simulink model "TosAeb"  (R2021a)
settings: Solver=FixedStepDiscrete  StartTime=0.0  StopTime=inf  FixedStep=0.01  RelTol=1e-3
undefined symbols (not defined in the file or in its folder): ACCModSts, AccSts1, AEB_ObjectMovingOrientation, AEB_ObjectMovingState, CN_BTCActive, CN_CurrentGearR, CN_CurrentGearR_DHT, CN_PTCActive, CN_VDCActive, CN_VSC_EspOff, CN_VSC_FCW_AEB_Error, CN_VSC_FCW_AEB_NoDispay, CN_VSC_FCW_AEB_PerfmDgdtn, CN_VSC_FCW_AEB_TempUnAvlbl, CN_VSC_VehStandstill, CNaccelAvoidMax, CNaccelAvoidMin, CNbrakePedalPress, CNcomfortStrLeftAccelMax, CNcomfortStrLeftAccelMin, CNcomfortStrRightAccelMax, CNcomfortStrRightAccelMin, CNDrvDoorOpen, CNDrvModeEco, CNDrvModeNorm, CNDrvModeOther, CNDrvModeSport, CNDrvSBRreminder, CNNull, CNobjInvalidID, CNPassDoorOpen, CNPassSBRreminder, CNPEBSAccelNegInf, CNPEBSAccelNull, CNPEBSTimeInf, CNPEBSTimeNull, CNSTOPMAX, CNSTOPNULL, CNsubjWidth, CNSwtOFF, CNSwtON, CNTTCMAX, CNTTCNULL, CNVehConfigAEBCycNone, CNVehConfigAEBNone, CNVehConfigAEBPedNone, CNVehConfigFCWNone, CNVehConfigJANone, CollisionSeverity, FALSE, FCWSnvty, JACycObjCalcInfoDefaultParams, JAPedObjCalcInfoDefaultParams, JAVehObjCalcInfoDefaultParams, latCycObjCalcInfoDefaultParams, latPedObjCalcInfoDefaultParams, longObjCalcInfoDefaultParams, ModSts, movingStatus, ObjectType ... (+1112)
libraries: simulink x3031, AEBSFunctionLib x50, Ta_Library x33, AEBSStateMachineLib x23, Mylibrary x12, simulink_extras x7, ObjSelect_lib x3, dspstat3 x2
(part 51 of 102)

== system TosAeb/AEB_Objsel/TA_Processor/PreProcessor/ObjectPrecessor/TAObjInfoCalc/For Each Subsystem/Info_Check1/Basic_Info_check/IDProcess ==
  ID_change : Inport
  ObsID_u8 : Inport  Port=2
  Compare To Constant : simulink/Logic and Bit Operations/Compare To Constant  relop===  const=1  OutDataTypeStr=boolean
  Compare To Constant1 : simulink/Logic and Bit Operations/Compare To Constant  relop=~=  const=0  OutDataTypeStr=boolean
  Constant : Constant  OutDataTypeStr=uint8
  Constant1 : Constant  Value=0  OutDataTypeStr=uint8
  Logical Operator : Logic  Operator=OR  AllPortsSameDT=off  OutDataTypeStr=boolean
  Switch : Switch  Criteria=u2 > Threshold
  Obs_ID : Outport
  wires:
  ID_change/1 -> Compare To Constant/1
  ObsID_u8/1 -> Compare To Constant1/1
  Compare To Constant/1 -> Logical Operator/1
  Compare To Constant1/1 -> Logical Operator/2
  Logical Operator/1 -> Switch/2
  Switch/1 -> Obs_ID/1
  Constant/1 -> Switch/1
  Constant1/1 -> Switch/3

== system TosAeb/AEB_Objsel/TA_Processor/PreProcessor/ObjectPrecessor/TAObjInfoCalc/For Each Subsystem/Info_Check1/Basic_Info_check/QuaCorrect ==
  Obj_ID : Inport
  ObsConfidence_u8 : Inport  Port=2
  Veh_AEBFunState : Inport  Port=3
  dy_flg : Inport  Port=4
  dx_flg : Inport  Port=5
  vx_flg : Inport  Port=6
  vy_flg : Inport  Port=7
  If1 : If
  Merge : Merge
  Subsystem : Subsystem
  quality_recrectionelse : Subsystem
  quality_recrectionif : Subsystem  TreatAsAtomicUnit=on
  Fus_ObjQuality : Outport
  wires:
  Merge/1 -> Fus_ObjQuality/1
  quality_recrectionelse/1(Fus_ObjQuality) -> Merge/2
  quality_recrectionif/1(Fus_ObjQuality) -> Merge/1
  If1/1 -> quality_recrectionif/Action
  If1/2 -> quality_recrectionelse/Action
  vy_flg/1 -> quality_recrectionelse/6(vy_flg)
  vx_flg/1 -> quality_recrectionelse/5(vx_flg)
  dx_flg/1 -> quality_recrectionelse/4(dx_flg)
  dy_flg/1 -> quality_recrectionelse/3(dy_flg)
  Veh_AEBFunState/1 -> quality_recrectionelse/2(Veh_AEBFunState)
  ObsConfidence_u8/1 -> quality_recrectionif/1(Fus_TargetQuality), quality_recrectionelse/1(Fus_TargetQuality)
  Subsystem/1(Obj_Invalid) -> If1/1
  Obj_ID/1 -> Subsystem/1(Obj_ID)

== system TosAeb/AEB_Objsel/TA_Processor/PreProcessor/ObjectPrecessor/TAObjInfoCalc/For Each Subsystem/Info_Check1/Basic_Info_check/VxCorrect ==
  Obj_ID : Inport
  Fus_ObjVx_Single : Inport  Port=2
  Fus_ObjVxStd : Inport  Port=3
  Fus_ObjDx_Single : Inport  Port=4
  Veh_AEBFunState : Inport  Port=5
  If : If
  If Action Subsystem : Subsystem  TreatAsAtomicUnit=on
  Merge : Merge
  Merge3 : Merge
  Subsystem : Subsystem
  Subsystem3 : Subsystem
  Fus_ObjVx : Outport
  Vx_CorrectFlg : Outport  Port=2
  wires:
  Merge3/1 -> Vx_CorrectFlg/1
  Subsystem3/2(Vx_CorrectFlg) -> Merge3/2
  If Action Subsystem/2(Vx_CorrectFlg) -> Merge3/1
  Merge/1 -> Fus_ObjVx/1
  Subsystem3/1(Fus_ObjVx) -> Merge/2
  If Action Subsystem/1(Fus_ObjVx) -> Merge/1
  If/2 -> Subsystem3/Action
  Veh_AEBFunState/1 -> Subsystem3/4(Veh_AEBFunState)
  Fus_ObjVxStd/1 -> Subsystem3/3(Fus_ObjVxStd)
  Fus_ObjDx_Single/1 -> Subsystem3/2(Fus_ObjDx_Single)
  Fus_ObjVx_Single/1 -> If Action Subsystem/1(Fus_ObjVx_Single), Subsystem3/1(Fus_ObjVx_Single)
  If/1 -> If Action Subsystem/Action
  Obj_ID/1 -> Subsystem/1(Obj_ID)
  Subsystem/1(Obj_Invalid) -> If/1

== system TosAeb/AEB_Objsel/TA_Processor/PreProcessor/ObjectPrecessor/TAObjInfoCalc/For Each Subsystem/Info_Check1/Basic_Info_check/VyCorrect ==
  Obj_ID : Inport
  Fus_ObjVy_Single : Inport  Port=2
  Fus_TargetState : Inport  Port=3
  Veh_YawRate : Inport  Port=4
  Fus_ObsVyStd : Inport  Port=5
  Fus_ObjDy_Single : Inport  Port=6
  Veh_AEBFunState : Inport  Port=7
  If : If
  If Action Subsystem : Subsystem  TreatAsAtomicUnit=on
  Merge : Merge
  Merge3 : Merge
  Subsystem : Subsystem
  Subsystem3 : Subsystem
  Fus_ObjVy : Outport
  Vy_CorrectFlg : Outport  Port=2
  wires:
  Fus_TargetState/1 -> Subsystem3/4(Fus_TargetState)
  Subsystem3/2(Vy_CorrectFlg) -> Merge3/2
  Merge3/1 -> Vy_CorrectFlg/1
  If Action Subsystem/2(Vy_CorrectFlg) -> Merge3/1
  Subsystem3/1(Fus_ObjVy) -> Merge/2
  Merge/1 -> Fus_ObjVy/1
  If Action Subsystem/1(Fus_ObjVy) -> Merge/1
  If/2 -> Subsystem3/Action
  If/1 -> If Action Subsystem/Action
  Veh_AEBFunState/1 -> Subsystem3/3(Veh_AEBFunState)
  Fus_ObjDy_Single/1 -> Subsystem3/2(Fus_ObjDy_Single)
  Fus_ObjVy_Single/1 -> If Action Subsystem/1(Fus_ObjVy_Single), Subsystem3/1(Fus_ObjVy_Single)
  Obj_ID/1 -> Subsystem/1(Obj_ID)
  Subsystem/1(Obj_Invalid) -> If/1
  Fus_ObsVyStd/1 -> Subsystem3/6(Fus_ObsVyStd)
  Veh_YawRate/1 -> Subsystem3/5(Veh_YawRate)

== system TosAeb/AEB_Objsel/TA_Processor/PreProcessor/ObjectPrecessor/TAObjInfoCalc/For Each Subsystem/Info_Check1/ID_check/IDValid_check ==
  note: ObsConfidence_u8:
      | 				 根据CNN检测计算出的障碍物存在
      | 				 概率，当连续>6时，意味着高conf
      | 				障碍物目标。
  note: ObsAge_u16:
      | 			这个障碍的帧龄是存在的，因为一些后
      | 			处理逻辑来确保跟踪状态和置信度，
      | 			在第一帧中可能不是“1”
  note: ObsValid_u8 :
      | 			0=invalid Obstacle(default value)
      | 			1=new valid (detected this frame)
      | 			2=older valid
  ObsID_u8 : Inport
  ObsValid_u8 : Inport  Port=2
  ObsAge_u16 : Inport  Port=3
  ObsConfidence_u8 : Inport  Port=4
  Action Port : ActionPort  ActionPortLabel=else
  Compare To Constant : simulink/Logic and Bit Operations/Compare To Constant  relop=>=  const=1  OutDataTypeStr=boolean
  Compare To Constant1 : simulink/Logic and Bit Operations/Compare To Constant  relop=>=  const=1  OutDataTypeStr=boolean
  Compare To Constant2 : simulink/Logic and Bit Operations/Compare To Constant  relop=>=  const=6  OutDataTypeStr=boolean
  Constant : Constant  Value=0  OutDataTypeStr=uint8
  Logical Operator : Logic  AllPortsSameDT=off  OutDataTypeStr=boolean
  Logical Operator1 : Logic  Operator=NOT  AllPortsSameDT=off  OutDataTypeStr=boolean
  Switch : Switch  Criteria=u2 ~= 0
  Terminator : Terminator
  Fus_ObjID : Outport
  ID_Change : Outport  Port=2
  wires:
  ObsValid_u8/1 -> Compare To Constant/1
  ObsAge_u16/1 -> Compare To Constant1/1
  ObsConfidence_u8/1 -> Compare To Constant2/1
  Compare To Constant/1 -> Logical Operator/1
  Compare To Constant1/1 -> Logical Operator/2
  Logical Operator/1 -> Switch/2, Logical Operator1/1
  ObsID_u8/1 -> Switch/1
  Constant/1 -> Switch/3
  Switch/1 -> Fus_ObjID/1
  Logical Operator1/1 -> ID_Change/1
  Compare To Constant2/1 -> Terminator/1

== system TosAeb/AEB_Objsel/TA_Processor/PreProcessor/ObjectPrecessor/TAObjInfoCalc/For Each Subsystem/Info_Check1/ID_check/ID_Judge ==
  ObsID_u8 : Inport
  Compare To Constant : simulink/Logic and Bit Operations/Compare To Constant  relop===  const=0  OutDataTypeStr=boolean
  ID_Invalid : Outport
  wires:
  ObsID_u8/1 -> Compare To Constant/1
  Compare To Constant/1 -> ID_Invalid/1

== system TosAeb/AEB_Objsel/TA_Processor/PreProcessor/ObjectPrecessor/TAObjInfoCalc/For Each Subsystem/Info_Check1/ID_check/Invalid ==
  ObsID_u8 : Inport
  Action Port : ActionPort  ActionPortLabel=if(u1 == 1)
  Constant : Constant  Value=0  OutDataTypeStr=boolean
  Signal Conversion : SignalConversion  OverrideOpt=off
  Fus_ObjID : Outport
  ID_Change : Outport  Port=2
  wires:
  ObsID_u8/1 -> Signal Conversion/1
  Signal Conversion/1 -> Fus_ObjID/1
  Constant/1 -> ID_Change/1

== system TosAeb/AEB_Objsel/TA_Processor/PreProcessor/ObjectPrecessor/TAObjInfoCalc/For Each Subsystem/Info_Check1/Orientation_check/Vyaverage ==
  ObsVelYAbs_s : Inport
  Abs{,1..4} (5 alike) : Abs
  Add : Sum  Inputs=+++++
  Constant : Constant  Value=0.2  OutDataTypeStr=single
  Delay : Delay  DelayLength=3
  Delay1 : Delay  DelayLength=4
  Delay2 : Delay  DelayLength=5
  Product : Product
  Unit Delay : UnitDelay  SampleTime=-1  HasFrameUpgradeWarning=on
  Obs_Vyaverage : Outport
  wires:
  Abs/1 -> Add/1
  ObsVelYAbs_s/1 -> Delay2/1, Delay1/1, Delay/1, Unit Delay/1, Abs/1
  Add/1 -> Product/1
  Constant/1 -> Product/2
  Product/1 -> Obs_Vyaverage/1
  Unit Delay/1 -> Abs1/1
  Abs1/1 -> Add/2
  Delay/1 -> Abs2/1
  Delay1/1 -> Abs3/1
  Delay2/1 -> Abs4/1
  Abs2/1 -> Add/3
  Abs3/1 -> Add/4
  Abs4/1 -> Add/5

== system TosAeb/AEB_Objsel/TA_Processor/PreProcessor/ObjectPrecessor/TAObjInfoCalc/For Each Subsystem/Info_Check1/State_check/Spd_C ==
  ObsVelXAbs_s : Inport
  ObsVelYAbs_s : Inport  Port=2
  Add : Sum
  Product : Product
  Product1 : Product
  Sqrt : Sqrt  SaturateOnIntegerOverflow=on
  Spd : Outport
  wires:
  ObsVelXAbs_s/1 -> Product/2, Product/1
  ObsVelYAbs_s/1 -> Product1/2, Product1/1
  Product/1 -> Add/1
  Product1/1 -> Add/2
  Add/1 -> Sqrt/1
  Sqrt/1 -> Spd/1

== system TosAeb/AEB_Objsel/TA_Processor/PreProcessor/ObjectPrecessor/TAObjInfoCalc/For Each Subsystem/Info_Check1/Type_Precheck/ObsSpd_Calculate ==
  ObsVelXAbs_s : Inport
  ObsVelYAbs_s : Inport  Port=2
  Add : Sum
  Product : Product
  Product1 : Product
  Sqrt : Sqrt  SaturateOnIntegerOverflow=on
  ObsSpd_s : Outport
  wires:
  ObsVelXAbs_s/1 -> Product/2, Product/1
  ObsVelYAbs_s/1 -> Product1/2, Product1/1
  Product/1 -> Add/1
  Product1/1 -> Add/2
  Add/1 -> Sqrt/1
  Sqrt/1 -> ObsSpd_s/1

== system TosAeb/AEB_Objsel/TA_Processor/PreProcessor/ObjectPrecessor/TAObjInfoCalc/For Each Subsystem/Info_Check1/Type_Process/Heading ==
  For_Number : Inport
  TA_ObjInfo : Inport  Port=2
  VehSelfData : Inport  Port=3
  Obj_AbsInfo : Subsystem
  HeadingAnlge : Outport
  Obj_AbsAccly : Outport  Port=2
  Obj_AbsSpdy : Outport  Port=3
  wires:
  TA_ObjInfo/1 -> Obj_AbsInfo/2(TA_ObjInfo)
  VehSelfData/1 -> Obj_AbsInfo/1(VehSelfData)
  Obj_AbsInfo/1(HeadingAnlge) -> HeadingAnlge/1
  Obj_AbsInfo/2(Obj_AbsAccly) -> Obj_AbsAccly/1
  Obj_AbsInfo/3(Obj_AbsSpdy) -> Obj_AbsSpdy/1
  For_Number/1 -> Obj_AbsInfo/3(For_Number)

== system TosAeb/FcwAebJa_Step_sys/ALG_AEBSystem/ALG_AEBController/AEB_FuncCondCalcApp/CycFunctionCondCalc/cycAEBfullSuppAborCond/cycAEBfullAborCondNoStandstillCalc/cycAEBfullSituationRelAborCondCalc/ObjLatMovContiInvalidCalc ==
  note: Unknown
  subjVel : Inport
  dy : Inport  Port=2
  dx : Inport  Port=3
  ObjectMovingState : Inport  Port=4
  Abs : Abs
  Abs1 : Abs
  Abs2 : Abs
  Compare To Constant : simulink/Logic and Bit Operations/Compare To Constant  relop=>  const=P_AEB_Func_cycAEBfullSubjVelThresObjLatMovContiInvalid  OutDataTypeStr=boolean
  Compare To Constant1 : simulink/Logic and Bit Operations/Compare To Constant  relop=>  const=0  OutDataTypeStr=boolean
  Compare To Constant2 : simulink/Logic and Bit Operations/Compare To Constant  relop===  const=AEB_ObjectMovingState.Stopped  OutDataTypeStr=boolean
  Compare To Constant3 : simulink/Logic and Bit Operations/Compare To Constant  relop===  const=AEB_ObjectMovingState.Stopped  OutDataTypeStr=boolean
  Compare To Constant4 : simulink/Logic and Bit Operations/Compare To Constant  relop=>  const=P_AEB_Func_cycAEBfullDyAbsThresObjLatMovContiInvalid  OutDataTypeStr=boolean
  Compare To Constant5 : simulink/Logic and Bit Operations/Compare To Constant  relop=>  const=P_AEB_Func_cycAEBfullAZAngleThresObjLatMovContiInvalid  OutDataTypeStr=boolean
  Logical Operator : Logic  AllPortsSameDT=off  OutDataTypeStr=boolean
  Logical Operator1 : Logic  Inputs=5  AllPortsSameDT=off  OutDataTypeStr=boolean
  Product : Product
  Trigonometric Function : Trigonometry  Operator=atan2
  Unit Delay : UnitDelay  InitialCondition=AEB_ObjectMovingState.UnknownState  SampleTime=-1  HasFrameUpgradeWarning=on
  dyFirstDetect : Ground
  ObjLatMovContiInvalid : Outport
  wires:
  Compare To Constant5/1 -> Logical Operator1/4
  dyFirstDetect/1 -> Product/1
  Logical Operator1/1 -> ObjLatMovContiInvalid/1
  Compare To Constant/1 -> Logical Operator1/1
  Compare To Constant1/1 -> Logical Operator1/2
  Compare To Constant4/1 -> Logical Operator1/3
  Trigonometric Function/1 -> Compare To Constant5/1
  Logical Operator/1 -> Logical Operator1/5
  Abs2/1 -> Compare To Constant4/1
  Compare To Constant2/1 -> Logical Operator/2
  Compare To Constant3/1 -> Logical Operator/1
  Unit Delay/1 -> Compare To Constant2/1
  ObjectMovingState/1 -> Unit Delay/1, Compare To Constant3/1
  Abs1/1 -> Trigonometric Function/2
  dx/1 -> Abs1/1
  Abs/1 -> Trigonometric Function/1
  dy/1 -> Product/2, Abs/1, Abs2/1
  Product/1 -> Compare To Constant1/1
  subjVel/1 -> Compare To Constant/1

== system TosAeb/FcwAebJa_Step_sys/ALG_AEBSystem/ALG_AEBController/AEB_FuncCondCalcApp/CycFunctionCondCalc/cycAEBfullSuppAborCond/cycAEBfullAborCondNoStandstillCalc/cycAEBfullSituationRelAborCondCalc/Subsystem ==
  cycCollisionSeverity : Inport
  cycVehVelReduced : Inport  Port=2
  objLatCollsionRiskProb : Inport  Port=3
  ObjLatMovContiInvalid : Inport  Port=4
  Compare To Constant : simulink/Logic and Bit Operations/Compare To Constant  relop===  const=CollisionSeverity.None  OutDataTypeStr=boolean
  Compare To Constant1 : simulink/Logic and Bit Operations/Compare To Constant  relop=>  const=P_AEB_Func_cycAEBfullVelReduceAborThres  OutDataTypeStr=boolean
  Compare To Constant2 : simulink/Logic and Bit Operations/Compare To Constant  relop===  const=TRUE  OutDataTypeStr=boolean
  Compare To Constant3 : simulink/Logic and Bit Operations/Compare To Constant  relop=<  const=P_AEB_Func_cycAEBfullColliisonRiskProbAborThres  OutDataTypeStr=boolean
  Logical Operator1 : Logic  Operator=OR  Inputs=4  AllPortsSameDT=off  OutDataTypeStr=boolean
  cycAEBfullSituationRelAborCondFulfilled : Outport
  wires:
  Compare To Constant2/1 -> Logical Operator1/4
  ObjLatMovContiInvalid/1 -> Compare To Constant2/1
  Compare To Constant1/1 -> Logical Operator1/2
  cycVehVelReduced/1 -> Compare To Constant1/1
  objLatCollsionRiskProb/1 -> Compare To Constant3/1
  Compare To Constant/1 -> Logical Operator1/1
  cycCollisionSeverity/1 -> Compare To Constant/1
  Compare To Constant3/1 -> Logical Operator1/3
  Logical Operator1/1 -> cycAEBfullSituationRelAborCondFulfilled/1

== system TosAeb/FcwAebJa_Step_sys/ALG_AEBSystem/ALG_AEBController/AEB_FuncCondCalcApp/CycFunctionCondCalc/cycAEBfullSuppAborCond/cycAEBfullSuppCondCalc/cycAEBfullSituationRelSuppCondCalc/DistanceBelowMinimumForFullBrakeCalc ==
  dxFrontBumper : Inport
  subVel : Inport  Port=2
  Constant : Constant  Value=P_AEB_Func_cycAEBfullLongDistTimeOffset
  Constant1 : Constant  Value=0  OutDataTypeStr=single
  Product : Product
  Relational Operator : RelationalOperator  Operator=<=  OutDataTypeStr=boolean  RndMeth=Simplest
  Terminator : Terminator
  distMinCond4Brake : Outport
  wires:
  Constant/1 -> Product/2
  subVel/1 -> Product/1
  dxFrontBumper/1 -> Relational Operator/1
  Relational Operator/1 -> distMinCond4Brake/1
  Product/1 -> Terminator/1
  Constant1/1 -> Relational Operator/2

== system TosAeb/FcwAebJa_Step_sys/ALG_AEBSystem/ALG_AEBController/AEB_FuncCondCalcApp/CycFunctionCondCalc/cycAEBfullSuppAborCond/cycAEBfullSuppCondCalc/cycAEBfullSituationRelSuppCondCalc/DistanceTriggerForBarkingCalc ==
  dxFrontBumper : Inport
  distBraking4FullBrake : Inport  Port=2
  distSecure : Inport  Port=3
  Add : Sum  Inputs=2
  Relational Operator : RelationalOperator  Operator=<=  OutDataTypeStr=boolean  RndMeth=Simplest
  objLongDistCond4FullBrake : Outport
  wires:
  Relational Operator/1 -> objLongDistCond4FullBrake/1
  distSecure/1 -> Add/2
  distBraking4FullBrake/1 -> Add/1
  Add/1 -> Relational Operator/2
  dxFrontBumper/1 -> Relational Operator/1

== system TosAeb/FcwAebJa_Step_sys/ALG_AEBSystem/ALG_AEBController/AEB_FuncCondCalcApp/CycFunctionCondCalc/cycAEBfullSuppAborCond/cycAEBfullSuppCondCalc/cycAEBfullSituationRelSuppCondCalc/LatDistCond4fullBrakeCalc ==
  dy : Inport
  FcwHMISetting : Inport  Port=2
  vy : Inport  Port=3
  LatDistCondCalc : Subsystem
  LatDistThresCalc : Subsystem
  latDistCond4fullBrake : Outport
  wires:
  dy/1 -> LatDistCondCalc/1(dy)
  FcwHMISetting/1 -> LatDistThresCalc/1(FcwHMISetting)
  vy/1 -> LatDistThresCalc/2(vy)
  LatDistCondCalc/1(latDistCond4fullBrake) -> latDistCond4fullBrake/1
  LatDistThresCalc/1(latDistThres) -> LatDistCondCalc/2(latDistThres)

== system TosAeb/FcwAebJa_Step_sys/ALG_AEBSystem/ALG_AEBController/AEB_FuncCondCalcApp/CycFunctionCondCalc/cycAEBfullSuppAborCond/cycAEBfullSuppCondCalc/cycAEBfullSituationRelSuppCondCalc/Subsystem ==
  latCollisionSeverity : Inport
  subjCurvTraj : Inport  Port=2
  subjLatAccel : Inport  Port=3
  cycCollisionRisk : Inport  Port=4
  cycCollisionProbFlg : Inport  Port=5
  ObjectTypeCameraConfirmed : Inport  Port=6
  objLatVyCond : Inport  Port=7
  latDistCond4fullBrake : Inport  Port=8
  distMinCond4Brake : Inport  Port=9
  objLongDistCond4FullBrake : Inport  Port=10
  Compare To Constant1 : simulink/Logic and Bit Operations/Compare To Constant  relop===  const=CollisionSeverity.None  OutDataTypeStr=boolean
  Compare To Constant{6..7,10..11} (4 alike) : simulink/Logic and Bit Operations/Compare To Constant  relop===  const=FALSE  OutDataTypeStr=boolean
  Compare To Constant12 : simulink/Logic and Bit Operations/Compare To Constant  relop=>=  const=P_AEB_Func_cycAEBfullColliisonRiskProbSupThres4Radar  OutDataTypeStr=boolean
  Compare To Constant13 : simulink/Logic and Bit Operations/Compare To Constant  relop=>=  const=P_AEB_Func_cycAEBfullColliisonRiskProbSupThres4Fusion  OutDataTypeStr=boolean
  Compare To Constant14 : simulink/Logic and Bit Operations/Compare To Constant  relop===  const=TRUE  OutDataTypeStr=boolean
  Compare To Constant2 : simulink/Logic and Bit Operations/Compare To Constant  relop=>  const=P_AEB_Func_cycAEBfullSubjCurvTrajSupThres  OutDataTypeStr=boolean
  Compare To Constant3 : simulink/Logic and Bit Operations/Compare To Constant  relop=>  const=P_AEB_Func_cycAEBfullSubjLatAccelSupThres  OutDataTypeStr=boolean
  Compare To Constant4 : simulink/Logic and Bit Operations/Compare To Constant  relop===  const=TRUE  OutDataTypeStr=boolean
  Compare To Constant5 : simulink/Logic and Bit Operations/Compare To Constant  relop===  const=CollisionSeverity.Low  OutDataTypeStr=boolean
  Compare To Constant8 : simulink/Logic and Bit Operations/Compare To Constant  relop===  const=CollisionSeverity.Medium  OutDataTypeStr=boolean
  Compare To Constant9 : simulink/Logic and Bit Operations/Compare To Constant  relop===  const=TRUE  OutDataTypeStr=boolean
  Logical Operator1 : Logic  Operator=OR  AllPortsSameDT=off  OutDataTypeStr=boolean
  Logical Operator2 : Logic  Operator=OR  Inputs=7  AllPortsSameDT=off  OutDataTypeStr=boolean
  Logical Operator3 : Logic  Operator=OR  Inputs=3  AllPortsSameDT=off  OutDataTypeStr=boolean
  Logical Operator4 : Logic  Operator=OR  AllPortsSameDT=off  OutDataTypeStr=boolean
  Switch : Switch  Criteria=u2 ~= 0
  cycAEBfullSituationRelSuppCondFulfilled : Outport
  wires:
  Compare To Constant10/1 -> Logical Operator4/2
  Compare To Constant6/1 -> Logical Operator4/1
  cycCollisionProbFlg/1 -> Compare To Constant10/1
  latDistCond4fullBrake/1 -> Compare To Constant9/1
  Compare To Constant8/1 -> Logical Operator3/3
  Compare To Constant5/1 -> Logical Operator3/2
  Compare To Constant1/1 -> Logical Operator3/1
  Compare To Constant3/1 -> Logical Operator1/2
  Compare To Constant2/1 -> Logical Operator1/1
  Logical Operator2/1 -> cycAEBfullSituationRelSuppCondFulfilled/1  "cycAEBfullSituationRelSuppCondFulfilled"
  subjCurvTraj/1 -> Compare To Constant2/1
  Logical Operator3/1 -> Logical Operator2/1
  Logical Operator1/1 -> Logical Operator2/2
  Logical Operator4/1 -> Logical Operator2/3
  subjLatAccel/1 -> Compare To Constant3/1
  objLatVyCond/1 -> Compare To Constant4/1
  objLongDistCond4FullBrake/1 -> Compare To Constant7/1
  latCollisionSeverity/1 -> Compare To Constant8/1, Compare To Constant5/1, Compare To Constant1/1
  Switch/1 -> Compare To Constant6/1
  distMinCond4Brake/1 -> Compare To Constant11/1
  Compare To Constant9/1 -> Logical Operator2/7  "AEB_CycLatDistSuppCond4FullBrake"
  Compare To Constant7/1 -> Logical Operator2/4  "AEB_CycLongDistSuppCond4FullBrake"
  Compare To Constant11/1 -> Logical Operator2/5  "AEB_CycLongDistMinSuppCond4FullBrake"
  Compare To Constant4/1 -> Logical Operator2/6  "AEB_CycLatVySuppCond4FullBrake"
  cycCollisionRisk/1 -> Compare To Constant12/1, Compare To Constant13/1
  ObjectTypeCameraConfirmed/1 -> Compare To Constant14/1
  Compare To Constant12/1 -> Switch/3
  Compare To Constant14/1 -> Switch/2
  Compare To Constant13/1 -> Switch/1

== system TosAeb/FcwAebJa_Step_sys/ALG_AEBSystem/ALG_AEBController/AEB_FuncCondCalcApp/CycFunctionCondCalc/cycAEBfullSuppAborCond/cycAEBfullSuppCondCalc/cycAEBfullSituationRelSuppCondCalc/objLatVyCond4FullBrakeCalc ==
  ObjectTypeCameraConfirmed : Inport
  vyCorr : Inport  Port=2
  Abs : Abs
  Compare To Constant1 : simulink/Logic and Bit Operations/Compare To Constant  relop=>  const=P_AEB_Func_cycAEBfullVyAbsMaxThres4Fusion  OutDataTypeStr=boolean
  Compare To Constant2 : simulink/Logic and Bit Operations/Compare To Constant  relop=<  const=P_AEB_Func_cycAEBfullVyAbsMinThres4Fusion  OutDataTypeStr=boolean
  Compare To Constant3 : simulink/Logic and Bit Operations/Compare To Constant  relop===  const=TRUE  OutDataTypeStr=boolean
  Compare To Constant4 : simulink/Logic and Bit Operations/Compare To Constant  relop=>  const=P_AEB_Func_cycAEBfullVyAbsMaxThres4Radar  OutDataTypeStr=boolean
  Compare To Constant5 : simulink/Logic and Bit Operations/Compare To Constant  relop=<  const=P_AEB_Func_cycAEBfullVyAbsMinThres4Radar  OutDataTypeStr=boolean
  Logical Operator : Logic  Operator=OR  AllPortsSameDT=off  OutDataTypeStr=boolean
  Logical Operator1 : Logic  Operator=OR  AllPortsSameDT=off  OutDataTypeStr=boolean
  Switch : Switch  Criteria=u2 ~= 0
  objLatVyCond : Outport
  wires:
  Abs/1 -> Compare To Constant4/1, Compare To Constant5/1, Compare To Constant1/1, Compare To Constant2/1
  Logical Operator1/1 -> Switch/3
  Compare To Constant5/1 -> Logical Operator1/1
  Compare To Constant4/1 -> Logical Operator1/2
  Logical Operator/1 -> Switch/1
  Switch/1 -> objLatVyCond/1
  Compare To Constant3/1 -> Switch/2
  ObjectTypeCameraConfirmed/1 -> Compare To Constant3/1
  Compare To Constant1/1 -> Logical Operator/2
  Compare To Constant2/1 -> Logical Operator/1
  vyCorr/1 -> Abs/1

== system TosAeb/FcwAebJa_Step_sys/ALG_AEBSystem/ALG_AEBController/AEB_FuncCondCalcApp/CycFunctionCondCalc/cycAEBfullSuppAborCond/cycAEBfullSuppCondCalc/cycAEBfullVehRelSuppCondCalc/brakeReleventSuppCond ==
  VehConfigIbooster : Inport
  ESP_MasterCylindBrakePressValid : Inport  Port=2
  ESP_MasterCylindBrakePress : Inport  Port=3
  Compare To Constant : simulink/Logic and Bit Operations/Compare To Constant  relop===  const=FALSE  OutDataTypeStr=boolean
  If : If  IfExpression=u1
  Merge : Merge
  brakeReleventSuppCond_ESP : Subsystem  VariantControl=(default)
  brakeReleventSuppCond_IBooster : Subsystem  VariantControl=P_AEB_PowerTrainConfig == 1
  brakeReleventSuppCond : Outport
  wires:
  ESP_MasterCylindBrakePress/1 -> brakeReleventSuppCond_ESP/2(ESP_MasterCylindBrakePress)
  ESP_MasterCylindBrakePressValid/1 -> brakeReleventSuppCond_ESP/1(ESP_MasterCylindBrakePressValid)
  Merge/1 -> brakeReleventSuppCond/1
  brakeReleventSuppCond_IBooster/1(brakeReleventSuppCond) -> Merge/2
  brakeReleventSuppCond_ESP/1(brakeReleventSuppCond) -> Merge/1
  If/2 -> brakeReleventSuppCond_IBooster/Action
  If/1 -> brakeReleventSuppCond_ESP/Action
  Compare To Constant/1 -> If/1
  VehConfigIbooster/1 -> Compare To Constant/1

== system TosAeb/FcwAebJa_Step_sys/ALG_AEBSystem/ALG_AEBController/AEB_FuncCondCalcApp/CycFunctionCondCalc/cycAEBpartSuppAborCond/cycAEBpartAborCondNoStandstillCalc/cycAEBpartSituationRelAborCondCalc/ObjLatMovContiInvalidCalc ==
  note: 连续两个周期进行可标定
  subjVel : Inport
  dy : Inport  Port=2
  dx : Inport  Port=3
  ObjectMovingState : Inport  Port=4
  Abs : Abs
  Abs1 : Abs
  Abs2 : Abs
  Compare To Constant : simulink/Logic and Bit Operations/Compare To Constant  relop=>  const=P_AEB_Func_cycAEBpartSubjVelThresObjLatMovContiInvalid  OutDataTypeStr=boolean
  Compare To Constant1 : simulink/Logic and Bit Operations/Compare To Constant  relop=>  const=0  OutDataTypeStr=boolean
  Compare To Constant2 : simulink/Logic and Bit Operations/Compare To Constant  relop===  const=AEB_ObjectMovingState.Stopped  OutDataTypeStr=boolean
  Compare To Constant3 : simulink/Logic and Bit Operations/Compare To Constant  relop===  const=AEB_ObjectMovingState.Stopped  OutDataTypeStr=boolean
  Compare To Constant4 : simulink/Logic and Bit Operations/Compare To Constant  relop=>  const=P_AEB_Func_cycAEBpartDyAbsThresObjLatMovContiInvalid  OutDataTypeStr=boolean
  Compare To Constant5 : simulink/Logic and Bit Operations/Compare To Constant  relop=>  const=P_AEB_Func_cycAEBpartAZAngleThresObjLatMovContiInvalid  OutDataTypeStr=boolean
  Constant : Constant  OutDataTypeStr=boolean
  Logical Operator : Logic  AllPortsSameDT=off  OutDataTypeStr=boolean
  Logical Operator1 : Logic  Inputs=5  AllPortsSameDT=off  OutDataTypeStr=boolean
  Product : Product
  Terminator : Terminator
  Trigonometric Function : Trigonometry  Operator=atan2
  Unit Delay : UnitDelay  InitialCondition=AEB_ObjectMovingState.UnknownState  SampleTime=-1  HasFrameUpgradeWarning=on
  dyFirstDetect : Ground
  ObjLatMovContiInvalid : Outport
  wires:
  Compare To Constant5/1 -> Logical Operator1/4
  Constant/1 -> Logical Operator1/2
  dyFirstDetect/1 -> Product/1
  Logical Operator1/1 -> ObjLatMovContiInvalid/1
  Compare To Constant/1 -> Logical Operator1/1
  Compare To Constant1/1 -> Terminator/1
  Compare To Constant4/1 -> Logical Operator1/3
  Trigonometric Function/1 -> Compare To Constant5/1
  Logical Operator/1 -> Logical Operator1/5
  Abs2/1 -> Compare To Constant4/1
  Compare To Constant2/1 -> Logical Operator/2
  Compare To Constant3/1 -> Logical Operator/1
  Unit Delay/1 -> Compare To Constant2/1
  ObjectMovingState/1 -> Unit Delay/1, Compare To Constant3/1
  Abs1/1 -> Trigonometric Function/2
  dx/1 -> Abs1/1
  Abs/1 -> Trigonometric Function/1
  dy/1 -> Abs/1, Abs2/1, Product/2
  Product/1 -> Compare To Constant1/1
  subjVel/1 -> Compare To Constant/1

== system TosAeb/FcwAebJa_Step_sys/ALG_AEBSystem/ALG_AEBController/AEB_FuncCondCalcApp/CycFunctionCondCalc/cycAEBpartSuppAborCond/cycAEBpartAborCondNoStandstillCalc/cycAEBpartSituationRelAborCondCalc/Subsystem ==
  latCollisionSeverity : Inport
  cycVehVelReduced : Inport  Port=2
  CollsionRiskProb : Inport  Port=3
  ObjLatMovContiInvalid : Inport  Port=4
  Compare To Constant : simulink/Logic and Bit Operations/Compare To Constant  relop===  const=CollisionSeverity.None  OutDataTypeStr=boolean
  Compare To Constant1 : simulink/Logic and Bit Operations/Compare To Constant  relop=>  const=P_AEB_Func_cycAEBpartVelReduceAborThres  OutDataTypeStr=boolean
  Compare To Constant2 : simulink/Logic and Bit Operations/Compare To Constant  relop===  const=TRUE  OutDataTypeStr=boolean
  Compare To Constant3 : simulink/Logic and Bit Operations/Compare To Constant  relop=<  const=P_AEB_Func_cycAEBpartCollisonRiskProbAborThres  OutDataTypeStr=boolean
  Logical Operator1 : Logic  Operator=OR  Inputs=4  AllPortsSameDT=off  OutDataTypeStr=boolean
  cycAEBpartSituationRelAborCondFulfilled : Outport
  wires:
  Compare To Constant2/1 -> Logical Operator1/4  "ObjLatMovContiInvalidRelAborCondFulfilled"
  ObjLatMovContiInvalid/1 -> Compare To Constant2/1
  Compare To Constant1/1 -> Logical Operator1/2  "cycVehVelReducedRelAborCondFulfilled"
  cycVehVelReduced/1 -> Compare To Constant1/1
  CollsionRiskProb/1 -> Compare To Constant3/1
  Compare To Constant/1 -> Logical Operator1/1  "latCollisionSeverityRelAborCondFulfilled"
  latCollisionSeverity/1 -> Compare To Constant/1
  Compare To Constant3/1 -> Logical Operator1/3  "CollsionRiskProbRelAborCondFulfilled"
  Logical Operator1/1 -> cycAEBpartSituationRelAborCondFulfilled/1

== system TosAeb/FcwAebJa_Step_sys/ALG_AEBSystem/ALG_AEBController/AEB_FuncCondCalcApp/CycFunctionCondCalc/cycAEBpartSuppAborCond/cycAEBpartSuppCondCalc/cycAEBpartSituationRelActiveCondCalc/DistanceBelowMinimumForFullBrakeCalc ==
  dxFrontBumper : Inport
  subVel : Inport  Port=2
  Constant : Constant  Value=P_AEB_Func_cycAEBpartLongDistTimeOffset
  Constant1 : Constant  Value=0.2  OutDataTypeStr=single
  Product : Product
  Relational Operator : RelationalOperator  Operator=<=  OutDataTypeStr=boolean  RndMeth=Simplest
  Terminator : Terminator
  distMinCond4Brake : Outport
  wires:
  Constant/1 -> Product/2
  subVel/1 -> Product/1
  dxFrontBumper/1 -> Relational Operator/1
  Relational Operator/1 -> distMinCond4Brake/1
  Product/1 -> Terminator/1
  Constant1/1 -> Relational Operator/2

== system TosAeb/FcwAebJa_Step_sys/ALG_AEBSystem/ALG_AEBController/AEB_FuncCondCalcApp/CycFunctionCondCalc/cycAEBpartSuppAborCond/cycAEBpartSuppCondCalc/cycAEBpartSituationRelActiveCondCalc/LatDistCond4PartBrakeCalc ==
  dy : Inport
  FcwHMISetting : Inport  Port=2
  vyCorr : Inport  Port=3
  LatDistCondCalc : Subsystem
  LatDistThresCalc : Subsystem
  latDistCond4PartBrake : Outport
  wires:
  vyCorr/1 -> LatDistThresCalc/2(vyCorr)
  LatDistThresCalc/1(latDistThres) -> LatDistCondCalc/2(latDistThres)
  LatDistCondCalc/1(latDistCond4PartBrake) -> latDistCond4PartBrake/1
  dy/1 -> LatDistCondCalc/1(dy)
  FcwHMISetting/1 -> LatDistThresCalc/1(FcwHMISetting)

== system TosAeb/FcwAebJa_Step_sys/ALG_AEBSystem/ALG_AEBController/AEB_FuncCondCalcApp/CycFunctionCondCalc/cycAEBpartSuppAborCond/cycAEBpartSuppCondCalc/cycAEBpartSituationRelActiveCondCalc/cycAEBpartSituationRelSuppCondCalc ==
  latCollisionSeverity : Inport
  subjCurvTraj : Inport  Port=2
  subjLatAccel : Inport  Port=3
  PreCollisionFlg : Inport  Port=4
  colliisonRiskProb : Inport  Port=5
  ObjectTypeCameraConfirmed : Inport  Port=6
  objLatVyCond : Inport  Port=7
  objLongDistCond4PartBrake : Inport  Port=8
  distMinCond4Brake : Inport  Port=9
  latDistCond4PartBrake : Inport  Port=10
  Compare To Constant1 : simulink/Logic and Bit Operations/Compare To Constant  relop===  const=CollisionSeverity.None  OutDataTypeStr=boolean
  Compare To Constant{4,6,8..10} (5 alike) : simulink/Logic and Bit Operations/Compare To Constant  relop===  const=FALSE  OutDataTypeStr=boolean
  Compare To Constant11 : simulink/Logic and Bit Operations/Compare To Constant  relop===  const=TRUE  OutDataTypeStr=boolean
  Compare To Constant12 : simulink/Logic and Bit Operations/Compare To Constant  relop=>=  const=P_AEB_Func_cycAEBpartColliisonRiskProbSupThres4Fusion  OutDataTypeStr=boolean
  Compare To Constant13 : simulink/Logic and Bit Operations/Compare To Constant  relop=>=  const=P_AEB_Func_cycAEBpartColliisonRiskProbSupThres4Radar  OutDataTypeStr=boolean
  Compare To Constant2 : simulink/Logic and Bit Operations/Compare To Constant  relop=>  const=P_AEB_Func_cycAEBpartSubjCurvTrajSupThres  OutDataTypeStr=boolean
  Compare To Constant3 : simulink/Logic and Bit Operations/Compare To Constant  relop=>  const=P_AEB_Func_cycAEBpartSubjLatAccelSupThres  OutDataTypeStr=boolean
  Compare To Constant5 : simulink/Logic and Bit Operations/Compare To Constant  relop===  const=CollisionSeverity.Low  OutDataTypeStr=boolean
  Compare To Constant7 : simulink/Logic and Bit Operations/Compare To Constant  relop===  const=TRUE  OutDataTypeStr=boolean
  Logical Operator1 : Logic  Operator=OR  AllPortsSameDT=off  OutDataTypeStr=boolean
  Logical Operator2 : Logic  Operator=OR  Inputs=8  AllPortsSameDT=off  OutDataTypeStr=boolean
  Logical Operator3 : Logic  Operator=OR  AllPortsSameDT=off  OutDataTypeStr=boolean
  Switch : Switch  Criteria=u2 ~= 0
  cycAEBpartSituationRelSuppCondFulfilled : Outport
  wires:
  Compare To Constant9/1 -> Logical Operator2/4  "colliisonFlgRelSuppCondFullfilled"
  Compare To Constant10/1 -> Logical Operator2/3  "colliisonRiskRelSuppCondFullfilled"
  Compare To Constant7/1 -> Logical Operator2/5  "AEB_CycLatVySuppCond4PartBrake"
  Compare To Constant4/1 -> Logical Operator2/8  "AEB_CycLatDistSuppCond4PartBrake"
  Compare To Constant8/1 -> Logical Operator2/7  "AEB_CycLongDistMinSuppCond4PartBrake"
  Compare To Constant6/1 -> Logical Operator2/6  "AEB_CycLongDistSuppCond4PartBrake"
  Compare To Constant13/1 -> Switch/3
  ObjectTypeCameraConfirmed/1 -> Compare To Constant11/1
  Compare To Constant12/1 -> Switch/1
  Compare To Constant11/1 -> Switch/2
  colliisonRiskProb/1 -> Compare To Constant12/1, Compare To Constant13/1
  Switch/1 -> Compare To Constant10/1
  PreCollisionFlg/1 -> Compare To Constant9/1
  distMinCond4Brake/1 -> Compare To Constant8/1
  Compare To Constant5/1 -> Logical Operator3/2
  Compare To Constant1/1 -> Logical Operator3/1
  objLongDistCond4PartBrake/1 -> Compare To Constant6/1
  latCollisionSeverity/1 -> Compare To Constant1/1, Compare To Constant5/1
  objLatVyCond/1 -> Compare To Constant7/1
  subjLatAccel/1 -> Compare To Constant3/1
  Logical Operator1/1 -> Logical Operator2/2  "subjMovingRelSuppCondFulfilled"
  Logical Operator3/1 -> Logical Operator2/1  "cycCollisionDgrLvlRelSuppCondFulfilled"
  subjCurvTraj/1 -> Compare To Constant2/1
  Logical Operator2/1 -> cycAEBpartSituationRelSuppCondFulfilled/1
  Compare To Constant2/1 -> Logical Operator1/1
  latDistCond4PartBrake/1 -> Compare To Constant4/1
  Compare To Constant3/1 -> Logical Operator1/2

== system TosAeb/FcwAebJa_Step_sys/ALG_AEBSystem/ALG_AEBController/AEB_FuncCondCalcApp/CycFunctionCondCalc/cycAEBpartSuppAborCond/cycAEBpartSuppCondCalc/cycAEBpartSituationRelActiveCondCalc/objLatVyCond4PrefillCalc ==
  ObjectTypeCameraConfirmed : Inport
  vy : Inport  Port=2
  Abs : Abs
  Compare To Constant1 : simulink/Logic and Bit Operations/Compare To Constant  relop=>  const=P_AEB_Func_cycAEBpartVyAbsMaxThres4Fusion  OutDataTypeStr=boolean
  Compare To Constant11 : simulink/Logic and Bit Operations/Compare To Constant  relop===  const=TRUE  OutDataTypeStr=boolean
  Compare To Constant2 : simulink/Logic and Bit Operations/Compare To Constant  relop=<  const=P_AEB_Func_cycAEBpartVyAbsMinThres4Fusion  OutDataTypeStr=boolean
  Compare To Constant3 : simulink/Logic and Bit Operations/Compare To Constant  relop=>  const=P_AEB_Func_cycAEBpartVyAbsMaxThres4Radar  OutDataTypeStr=boolean
  Compare To Constant4 : simulink/Logic and Bit Operations/Compare To Constant  relop=<  const=P_AEB_Func_cycAEBpartVyAbsMinThres4Radar  OutDataTypeStr=boolean
  Logical Operator : Logic  Operator=OR  AllPortsSameDT=off  OutDataTypeStr=boolean
  Logical Operator1 : Logic  Operator=OR  AllPortsSameDT=off  OutDataTypeStr=boolean
  Switch : Switch  Criteria=u2 ~= 0
  objLatVyCond : Outport
  wires:
  Abs/1 -> Compare To Constant3/1, Compare To Constant4/1, Compare To Constant1/1, Compare To Constant2/1
  vy/1 -> Abs/1
  Logical Operator1/1 -> Switch/3
  Compare To Constant4/1 -> Logical Operator1/1
  Compare To Constant3/1 -> Logical Operator1/2
  Logical Operator/1 -> Switch/1
  Switch/1 -> objLatVyCond/1
  Compare To Constant11/1 -> Switch/2
  ObjectTypeCameraConfirmed/1 -> Compare To Constant11/1
  Compare To Constant1/1 -> Logical Operator/2
  Compare To Constant2/1 -> Logical Operator/1

== system TosAeb/FcwAebJa_Step_sys/ALG_AEBSystem/ALG_AEBController/AEB_FuncCondCalcApp/CycFunctionCondCalc/cycAEBpartSuppAborCond/cycAEBpartSuppCondCalc/cycAEBpartSituationRelActiveCondCalc/objLongDistCond4PartBrakeCalc ==
  dxFrontBumper : Inport
  distBraking4PartBrake : Inport  Port=2
  distSecure : Inport  Port=3
  Add : Sum  Inputs=2
  Relational Operator : RelationalOperator  Operator=<=  OutDataTypeStr=boolean  RndMeth=Simplest
  objLongDistCond4PartBrake : Outport
  wires:
  distSecure/1 -> Add/2
  distBraking4PartBrake/1 -> Add/1
  Add/1 -> Relational Operator/2
  dxFrontBumper/1 -> Relational Operator/1
  Relational Operator/1 -> objLongDistCond4PartBrake/1

== system TosAeb/FcwAebJa_Step_sys/ALG_AEBSystem/ALG_AEBController/AEB_FuncCondCalcApp/CycFunctionCondCalc/cycAEBpartSuppAborCond/cycAEBpartSuppCondCalc/cycAEBpartVehRelActiveCondCalc/brakeReleventSuppCond ==
  VehConfigIbooster : Inport
  ESP_MasterCylindBrakePressValid : Inport  Port=2
  ESP_MasterCylindBrakePress : Inport  Port=3
  IB_BrkPedalAppld : Inport  Port=4
  Compare To Constant : simulink/Logic and Bit Operations/Compare To Constant  relop===  const=FALSE  OutDataTypeStr=boolean
  If : If  IfExpression=u1
  Merge : Merge
  brakeReleventSuppCond_ESP : Subsystem  VariantControl=(default)
  brakeReleventSuppCond_IBooster : Subsystem  VariantControl=P_AEB_PowerTrainConfig == 1
  brakeReleventSuppCond : Outport
  wires:
  ESP_MasterCylindBrakePress/1 -> brakeReleventSuppCond_ESP/2(ESP_MasterCylindBrakePress)
  ESP_MasterCylindBrakePressValid/1 -> brakeReleventSuppCond_ESP/1(ESP_MasterCylindBrakePressValid)
  IB_BrkPedalAppld/1 -> brakeReleventSuppCond_IBooster/1(IB_BrkPedalAppld)
  Merge/1 -> brakeReleventSuppCond/1
  brakeReleventSuppCond_IBooster/1(brakeReleventSuppCond) -> Merge/2
  brakeReleventSuppCond_ESP/1(brakeReleventSuppCond) -> Merge/1
  If/2 -> brakeReleventSuppCond_IBooster/Action
  If/1 -> brakeReleventSuppCond_ESP/Action
  Compare To Constant/1 -> If/1
  VehConfigIbooster/1 -> Compare To Constant/1

== system TosAeb/FcwAebJa_Step_sys/ALG_AEBSystem/ALG_AEBController/AEB_FuncCondCalcApp/CycFunctionCondCalc/cycPrefillSuppAborCond/cycPrefillSuppCondCalc/cycPrefillSituationRelSuppCondCalc/DistBrkCond4PrefillCalc ==
  dxFrontBumper : Inport
  disBraking4PartBrake : Inport  Port=2
  distSecure : Inport  Port=3
  subjVel : Inport  Port=4
  Add1 : Sum  Inputs=+++
  Constant : Constant  Value=P_AEB_Func_cycPrefillLongDistTimeOffset
  Product : Product
  Relational Operator : RelationalOperator  Operator=<=  OutDataTypeStr=boolean  RndMeth=Simplest
  distBrkCond4Prefill : Outport
  wires:
  Relational Operator/1 -> distBrkCond4Prefill/1
  Add1/1 -> Relational Operator/2
  dxFrontBumper/1 -> Relational Operator/1
  distSecure/1 -> Add1/2
  disBraking4PartBrake/1 -> Add1/1
  subjVel/1 -> Product/1
  Constant/1 -> Product/2
  Product/1 -> Add1/3

== system TosAeb/FcwAebJa_Step_sys/ALG_AEBSystem/ALG_AEBController/AEB_FuncCondCalcApp/CycFunctionCondCalc/cycPrefillSuppAborCond/cycPrefillSuppCondCalc/cycPrefillSituationRelSuppCondCalc/cycPrefillSituationRelSuppCondCalc ==
  latCollisionSeverity : Inport
  subjCurvTraj : Inport  Port=2
  subjLatAccel : Inport  Port=3
  colliisonRiskProb : Inport  Port=4
  ObjectTypeCameraConfirmed : Inport  Port=5
  objLatVyCond : Inport  Port=6
  objLongDistCond4Prefill : Inport  Port=7
  Compare To Constant1 : simulink/Logic and Bit Operations/Compare To Constant  relop===  const=CollisionSeverity.None  OutDataTypeStr=boolean
  Compare To Constant2 : simulink/Logic and Bit Operations/Compare To Constant  relop=>  const=P_AEB_Func_cycPrefillSubjCurvTrajSupThres  OutDataTypeStr=boolean
  Compare To Constant3 : simulink/Logic and Bit Operations/Compare To Constant  relop=>  const=P_AEB_Func_cycPrefillSubjLatAccelSupThres  OutDataTypeStr=boolean
  Compare To Constant4 : simulink/Logic and Bit Operations/Compare To Constant  relop===  const=TRUE  OutDataTypeStr=boolean
  Compare To Constant5 : simulink/Logic and Bit Operations/Compare To Constant  relop===  const=TRUE  OutDataTypeStr=boolean
  Compare To Constant6 : simulink/Logic and Bit Operations/Compare To Constant  relop===  const=FALSE  OutDataTypeStr=boolean
  Compare To Constant7 : simulink/Logic and Bit Operations/Compare To Constant  relop===  const=FALSE  OutDataTypeStr=boolean
  Compare To Constant8 : simulink/Logic and Bit Operations/Compare To Constant  relop=>  const=P_AEB_Func_cycPrefillColliisonRiskProbSupThres4Fusion  OutDataTypeStr=boolean
  Compare To Constant9 : simulink/Logic and Bit Operations/Compare To Constant  relop=>  const=P_AEB_Func_cycPrefillColliisonRiskProbSupThres4Radar  OutDataTypeStr=boolean
  Logical Operator1 : Logic  Operator=OR  AllPortsSameDT=off  OutDataTypeStr=boolean
  Logical Operator2 : Logic  Operator=OR  Inputs=5  AllPortsSameDT=off  OutDataTypeStr=boolean
  Switch : Switch  Criteria=u2 ~= 0
  cycPrefillSituationRelSuppCondFulfilled : Outport
  wires:
  Compare To Constant9/1 -> Switch/3
  Compare To Constant8/1 -> Switch/1
  Compare To Constant5/1 -> Switch/2
  ObjectTypeCameraConfirmed/1 -> Compare To Constant5/1
  colliisonRiskProb/1 -> Compare To Constant9/1, Compare To Constant8/1
  Compare To Constant7/1 -> Logical Operator2/5  "AEB_CycLongDistSuppCond4Prefill"
  Compare To Constant4/1 -> Logical Operator2/4  "AEB_CycLatVySuppCond4Prefill"
  Switch/1 -> Compare To Constant6/1
  latCollisionSeverity/1 -> Compare To Constant1/1
  objLongDistCond4Prefill/1 -> Compare To Constant7/1
  subjLatAccel/1 -> Compare To Constant3/1
  Compare To Constant6/1 -> Logical Operator2/3  "colliisonRiskCond4PrefillRelActiveCondFulfilled"
  Logical Operator1/1 -> Logical Operator2/2  "subjCurvTrajRelActiveCondFulfilled"
  Compare To Constant1/1 -> Logical Operator2/1  "latCollisionSeverityRelSuppCondFulfilled"
  subjCurvTraj/1 -> Compare To Constant2/1
  Logical Operator2/1 -> cycPrefillSituationRelSuppCondFulfilled/1  "cycPrefillSituationRelSuppCondFulfilled"
  Compare To Constant2/1 -> Logical Operator1/1
  objLatVyCond/1 -> Compare To Constant4/1
  Compare To Constant3/1 -> Logical Operator1/2

== system TosAeb/FcwAebJa_Step_sys/ALG_AEBSystem/ALG_AEBController/AEB_FuncCondCalcApp/CycFunctionCondCalc/cycPrefillSuppAborCond/cycPrefillSuppCondCalc/cycPrefillSituationRelSuppCondCalc/objLatVyCond4PrefillCalc ==
  ObjectTypeCameraConfirmed : Inport
  vy : Inport  Port=2
  Abs : Abs
  Compare To Constant1 : simulink/Logic and Bit Operations/Compare To Constant  relop=>  const=P_AEB_Func_cycPrefillVyAbsMaxThres4Fusion  OutDataTypeStr=boolean
  Compare To Constant2 : simulink/Logic and Bit Operations/Compare To Constant  relop=<  const=P_AEB_Func_cycPrefillVyAbsMinThres4Fusion  OutDataTypeStr=boolean
  Compare To Constant3 : simulink/Logic and Bit Operations/Compare To Constant  relop=>  const=P_AEB_Func_cycPrefillVyAbsMaxThres4Radar  OutDataTypeStr=boolean
  Compare To Constant4 : simulink/Logic and Bit Operations/Compare To Constant  relop=<  const=P_AEB_Func_cycPrefillVyAbsMinThres4Radar  OutDataTypeStr=boolean
  Compare To Constant5 : simulink/Logic and Bit Operations/Compare To Constant  relop===  const=TRUE  OutDataTypeStr=boolean
  Logical Operator : Logic  Operator=OR  AllPortsSameDT=off  OutDataTypeStr=boolean
  Logical Operator1 : Logic  Operator=OR  AllPortsSameDT=off  OutDataTypeStr=boolean
  Switch : Switch  Criteria=u2 ~= 0
  objLatVyCond : Outport
  wires:
  Abs/1 -> Compare To Constant2/1, Compare To Constant1/1, Compare To Constant4/1, Compare To Constant3/1
  Logical Operator1/1 -> Switch/3
  vy/1 -> Abs/1
  Compare To Constant4/1 -> Logical Operator1/1
  Compare To Constant3/1 -> Logical Operator1/2
  Switch/1 -> objLatVyCond/1
  Compare To Constant5/1 -> Switch/2
  ObjectTypeCameraConfirmed/1 -> Compare To Constant5/1
  Logical Operator/1 -> Switch/1
  Compare To Constant1/1 -> Logical Operator/2
  Compare To Constant2/1 -> Logical Operator/1

== system TosAeb/FcwAebJa_Step_sys/ALG_AEBSystem/ALG_AEBController/AEB_FuncCondCalcApp/CycFunctionCondCalc/cycPrefillSuppAborCond/cycPrefillSuppCondCalc/cycPrefillVehRelSuppCondCalc/brakeReleventSuppCond ==
  VehConfigIbooster : Inport
  ESP_MasterCylindBrakePressValid : Inport  Port=2
  ESP_MasterCylindBrakePress : Inport  Port=3
  IB_BrkPedalAppld : Inport  Port=4
  Compare To Constant : simulink/Logic and Bit Operations/Compare To Constant  relop===  const=FALSE  OutDataTypeStr=boolean
  If : If  IfExpression=u1
  Merge : Merge
  brakeReleventSuppCond_ESP : Subsystem  VariantControl=(default)
  brakeReleventSuppCond_IBooster : Subsystem  VariantControl=P_AEB_PowerTrainConfig == 1
  brakeReleventSuppCond : Outport
  wires:
  ESP_MasterCylindBrakePress/1 -> brakeReleventSuppCond_ESP/2(ESP_MasterCylindBrakePress)
  ESP_MasterCylindBrakePressValid/1 -> brakeReleventSuppCond_ESP/1(ESP_MasterCylindBrakePressValid)
  IB_BrkPedalAppld/1 -> brakeReleventSuppCond_IBooster/1(IB_BrkPedalAppld)
  Merge/1 -> brakeReleventSuppCond/1
  brakeReleventSuppCond_IBooster/1(brakeReleventSuppCond) -> Merge/2
  brakeReleventSuppCond_ESP/1(brakeReleventSuppCond) -> Merge/1
  If/2 -> brakeReleventSuppCond_IBooster/Action
  If/1 -> brakeReleventSuppCond_ESP/Action
  Compare To Constant/1 -> If/1
  VehConfigIbooster/1 -> Compare To Constant/1

== system TosAeb/FcwAebJa_Step_sys/ALG_AEBSystem/ALG_AEBController/AEB_FuncCondCalcApp/JaCycFunctionCondCalc/JaCycAEBfullSuppAborCond/JaCycAEBfullSuppCondCalc/JaCycAEBfullSuppVehCondCalc/brakeReleventSuppCond ==
  VehConfigIbooster : Inport
  ESP_MasterCylindBrakePress : Inport  Port=2
  Compare To Constant : simulink/Logic and Bit Operations/Compare To Constant  relop===  const=FALSE  OutDataTypeStr=boolean
  If : If  IfExpression=u1
  Merge : Merge
  brakeReleventSuppCond_ESP : Subsystem  VariantControl=(default)
  brakeReleventSuppCond_IBooster : Subsystem  VariantControl=P_AEB_PowerTrainConfig == 1
  brakeReleventSuppCond : Outport
  wires:
  VehConfigIbooster/1 -> Compare To Constant/1
  Compare To Constant/1 -> If/1
  If/1 -> brakeReleventSuppCond_ESP/Action
  If/2 -> brakeReleventSuppCond_IBooster/Action
  brakeReleventSuppCond_ESP/1(brakeReleventSuppCond) -> Merge/1
  brakeReleventSuppCond_IBooster/1(brakeReleventSuppCond) -> Merge/2
  Merge/1 -> brakeReleventSuppCond/1
  ESP_MasterCylindBrakePress/1 -> brakeReleventSuppCond_ESP/1(ESP_MasterCylindBrakePress)

== system TosAeb/FcwAebJa_Step_sys/ALG_AEBSystem/ALG_AEBController/AEB_FuncCondCalcApp/JaCycFunctionCondCalc/JaCycPrefillSuppAborCond/JaCycPrefillSuppCondCalc/JaCycPrefillSuppVehCondCalc/brakeReleventSuppCond ==
  VehConfigIbooster : Inport
  BrakePedalStatus_G : Inport  Port=2
  ESP_MasterCylindBrakePress : Inport  Port=3
  IB_BrkPedalAppld : Inport  Port=4
  Compare To Constant : simulink/Logic and Bit Operations/Compare To Constant  relop===  const=FALSE  OutDataTypeStr=boolean
  If : If  IfExpression=u1
  Merge : Merge
  brakeReleventSuppCond_ESP : Subsystem  VariantControl=(default)
  brakeReleventSuppCond_IBooster : Subsystem  VariantControl=P_AEB_PowerTrainConfig == 1
  brakeReleventSuppCond : Outport
  wires:
  VehConfigIbooster/1 -> Compare To Constant/1
  Compare To Constant/1 -> If/1
  If/1 -> brakeReleventSuppCond_ESP/Action
  If/2 -> brakeReleventSuppCond_IBooster/Action
  brakeReleventSuppCond_ESP/1(brakeReleventSuppCond) -> Merge/1
  brakeReleventSuppCond_IBooster/1(brakeReleventSuppCond) -> Merge/2
  Merge/1 -> brakeReleventSuppCond/1
  IB_BrkPedalAppld/1 -> brakeReleventSuppCond_IBooster/1(IB_BrkPedalAppld)
  ESP_MasterCylindBrakePress/1 -> brakeReleventSuppCond_ESP/2(ESP_MasterCylindBrakePress)
  BrakePedalStatus_G/1 -> brakeReleventSuppCond_ESP/1(BrakePedalStatus_G)

== system TosAeb/FcwAebJa_Step_sys/ALG_AEBSystem/ALG_AEBController/AEB_FuncCondCalcApp/JaPedFunctionCondCalc/AEBJAPedAEBfullSuppAborCond/AEBJAPedAEBfullSuppCondCalc/AEBJAPedAEBfullSuppVehCondCalc/brakeReleventSuppCond ==
  VehConfigIbooster : Inport
  ESP_MasterCylindBrakePress : Inport  Port=2
  Compare To Constant : simulink/Logic and Bit Operations/Compare To Constant  relop===  const=FALSE  OutDataTypeStr=boolean
  If : If  IfExpression=u1
  Merge : Merge
  brakeReleventSuppCond_ESP : Subsystem  VariantControl=(default)
  brakeReleventSuppCond_IBooster : Subsystem  VariantControl=P_AEB_PowerTrainConfig == 1
  brakeReleventSuppCond : Outport
  wires:
  ESP_MasterCylindBrakePress/1 -> brakeReleventSuppCond_ESP/1(ESP_MasterCylindBrakePress)
  Merge/1 -> brakeReleventSuppCond/1
  brakeReleventSuppCond_IBooster/1(brakeReleventSuppCond) -> Merge/2
  brakeReleventSuppCond_ESP/1(brakeReleventSuppCond) -> Merge/1
  If/2 -> brakeReleventSuppCond_IBooster/Action
  If/1 -> brakeReleventSuppCond_ESP/Action
  Compare To Constant/1 -> If/1
  VehConfigIbooster/1 -> Compare To Constant/1
